simulink model "l8_ver100"  (Simulink 7.9)
settings: Solver=FixedStepDiscrete  SolverMode=SingleTasking  StartTime=0.0  StopTime=10  RelTol=1e-3
undefined symbols (not defined in the file): sfp
libraries: xbsIndex_r4 x17119, xps_library x133, casper_library_flow_control x73, casper_library_misc x61, dspsigops x32
(part 5 of 159)

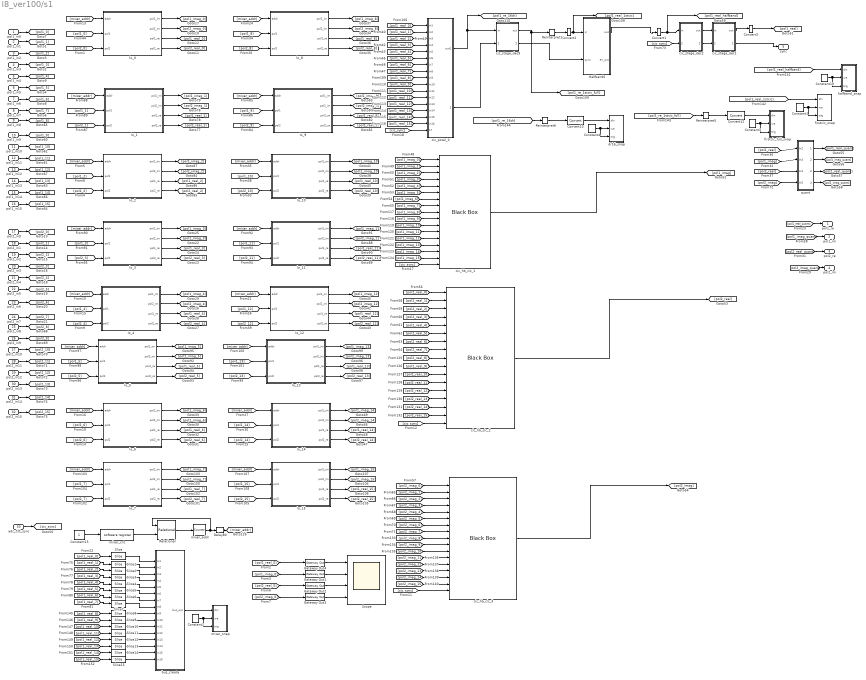
== system l8_ver100/s1 ==
  pol1_in0 : Inport
  pol1_in1 : Inport  Port=2
  pol1_in2 : Inport  Port=3
  pol1_in3 : Inport  Port=4
  pol1_in4 : Inport  Port=5
  pol1_in5 : Inport  Port=6
  pol1_in6 : Inport  Port=7
  pol1_in7 : Inport  Port=8
  pol1_in8 : Inport  Port=9
  pol1_in9 : Inport  Port=10
  pol1_in10 : Inport  Port=11
  pol1_in11 : Inport  Port=12
  pol1_in12 : Inport  Port=13
  pol1_in13 : Inport  Port=14
  pol1_in14 : Inport  Port=15
  pol1_in15 : Inport  Port=16
  pol2_in0 : Inport  Port=17
  pol2_in1 : Inport  Port=18
  pol2_in2 : Inport  Port=19
  pol2_in3 : Inport  Port=20
  pol2_in4 : Inport  Port=21
  pol2_in5 : Inport  Port=22
  pol2_in6 : Inport  Port=23
  pol2_in7 : Inport  Port=24
  pol2_in8 : Inport  Port=25
  pol2_in9 : Inport  Port=26
  pol2_in10 : Inport  Port=27
  pol2_in11 : Inport  Port=28
  pol2_in12 : Inport  Port=29
  pol2_in13 : Inport  Port=30
  pol2_in14 : Inport  Port=31
  pol2_in15 : Inport  Port=32
  adc_CIC_sync : Inport  Port=33
  Constant{1..4,19} (5 alike) : xbsIndex_r4/Constant  const=1  gui_display_data_type=Boolean  arith_type=Boolean  n_bits=32  bin_pt=0  preci_type=Single  exp_width=8  frac_width=24  explicit_period=on  period=1  equ=P=C  opselect=C  inp2=PCIN>>17  opr=+  inp1=P  carry=CIN  dbl_ovrd=off  block_type=constant
      settings: block_version=11.4
  Constant15 : Constant
  Convert1 : xbsIndex_r4/Convert  gui_display_data_type=Fixed-point  arith_type="Signed  (2's comp)"  n_bits=32  bin_pt=12  float_type=Single  exp_bits=8  fraction_bits=24  quantization=Truncate  overflow=Wrap  en=off  latency=1  dbl_ovrd=off  pipeline=off  block_type=convert
  Convert12 : xbsIndex_r4/Convert  gui_display_data_type=Fixed-point  arith_type="Signed  (2's comp)"  n_bits=64  bin_pt=0  float_type=Single  exp_bits=8  fraction_bits=24  quantization=Truncate  overflow=Wrap  en=off  latency=1  dbl_ovrd=off  pipeline=off  block_type=convert
  Convert13 : xbsIndex_r4/Convert  gui_display_data_type=Fixed-point  arith_type="Signed  (2's comp)"  n_bits=64  bin_pt=0  float_type=Single  exp_bits=8  fraction_bits=24  quantization=Truncate  overflow=Wrap  en=off  latency=1  dbl_ovrd=off  pipeline=off  block_type=convert
  Convert2 : xbsIndex_r4/Convert  gui_display_data_type=Fixed-point  arith_type="Signed  (2's comp)"  n_bits=32  bin_pt=18  float_type=Single  exp_bits=8  fraction_bits=24  quantization=Truncate  overflow=Wrap  en=off  latency=1  dbl_ovrd=off  pipeline=off  block_type=convert
  Convert3 : xbsIndex_r4/Convert  gui_display_data_type=Fixed-point  arith_type="Signed  (2's comp)"  n_bits=32  bin_pt=6  float_type=Single  exp_bits=8  fraction_bits=24  quantization=Truncate  overflow=Wrap  en=off  latency=1  dbl_ovrd=off  pipeline=off  block_type=convert
  Delay80 : xbsIndex_r4/Delay  rst=off  infoeditControl=Selection of Reset will increase slice count due to use of real FFs and instead of SRLs  en=off  latency=2  dbl_ovrd=off  reg_retiming=on  block_type=delay
      settings: block_version=11.4
  Gateway Out{1..4} (4 alike) : xbsIndex_r4/Gateway Out  inherit_from_input=off  hdl_port=on  timing_constraint=None  locs_specified=off  LOCs={}  UseAsDAC=off  DACChannel='1'  block_type=gatewayout
      settings: infoedit="Gateway out block.  Converts Xilinx fixed point or floating-point type inputs into ouputs of type Simulink integer, double, or fixed point.<br><br>Hardware notes:  In hardware these blocks become top level output ports or are discarded, depending on how they are configured."
  Halfband0 : Subsystem  AncestorBlock=gbt_library/Halfband  mask(Halfband FIR){mult_latency=3, add_latency=1, hold_period=2, passband=0.4, fir_len=7}
      mask prompts: mult_latency "Multiplication latency"; add_latency "Add latency"; hold_period "Hold Period"; passband "Width of Halfband passband (<0.5)"; fir_len "FIR Length (odd) [Number of Taps will be: 2*fir_len+1]"
      mask help: A halfband FIR for low rate computation.
      mask init: same as l8_ver100/s0/Halfband0
      settings: AttributesFormatString=|
      | 15-tap FIR filter (9 nonzero tap)
      |  hold period: 2
      |  mult latency: 3
      |  add latency: 1
  Reinterpret1 : xbsIndex_r4/Reinterpret  force_arith_type=off  arith_type="Signed  (2's comp)"  force_bin_pt=on  bin_pt=30  block_type=reinterpret
  Reinterpret5 : xbsIndex_r4/Reinterpret  force_arith_type=off  arith_type="Signed  (2's comp)"  force_bin_pt=on  bin_pt=0  block_type=reinterpret
  Reinterpret6 : xbsIndex_r4/Reinterpret  force_arith_type=off  arith_type="Signed  (2's comp)"  force_bin_pt=on  bin_pt=0  block_type=reinterpret
  Relational : xbsIndex_r4/Relational  mode=a=b  en=off  latency=1  dbl_ovrd=off  block_type=relational
  Scope : Scope  NumInputPorts=4
      settings: YMin=-0.0075~-1~-0.0075~-1  YMax=0.0075~1~0.0075~1
  Slice{,1..15} (16 alike) : xbsIndex_r4/Slice  nbits=8  boolean_output=off  mode=Upper Bit Location + Width  bit1=0  base1=MSB of Input  bit0=0  base0=LSB of Input  dbl_ovrd=off  block_type=slice
  bus_create : Subsystem  AncestorBlock=casper_library_flow_control/bus_create  mask(bus_create){inputNum=16}
      mask prompts: inputNum "Number of inputs:"
      mask init:
      | bus_create_init(gcb,...
      |     'inputNum', inputNum);
  cic_hb_cic_1 : xbsIndex_r4/Black Box  init_code=cichbcic_core_config  sim_method=Inactive  verbose=off  block_type=blackbox2
  cic_hb_cic_2 : xbsIndex_r4/Black Box  init_code=cichbcic_core_config  sim_method=Inactive  verbose=off  block_type=blackbox2
  cic_hb_cic_3 : xbsIndex_r4/Black Box  init_code=cichbcic_core_config  sim_method=Inactive  verbose=off  block_type=blackbox2
  cic_pow2_0 : Subsystem  AncestorBlock=gbt_library/cic_pow2  mask(CIC (pow2)){n_stages=4, dec_rate=16, input_clk_period=1, n_inputs=16, add_latency=1, n_bits=24, bin_pt=22}
      mask prompts: n_stages "CIC filter Order"; dec_rate "Decimation rate"; input_clk_period "Input Clock Period"; n_inputs "Number of inputs"; add_latency "Add latency"; n_bits "Input bit width"; bin_pt "Binary point"
      mask help:
      | A non-recursive CIC filter with parallel inputs.
      | Supports decimation rate of power of 2.
      | A multi-stage implementation with each stage of decimation rate 2.
      mask init: same as l8_ver100/s0/cic_pow2_0
      settings: AttributesFormatString=|
      | dec_rate = 16
      | Order = 4
  cic_stage_dec1 : Subsystem  AncestorBlock=gbt_library/cic_stage_dec2  mask(CIC stage (dec2)){n_stages=3, recursive=off, dec_rate=2, n_inputs=1, input_clk_period=1, input_period=4, add_latency=2, n_bits=35, bin_pt=12}
      mask prompts: n_stages "CIC filter Order"; recursive "Recursive?"; dec_rate "Decimation rate"; n_inputs "Number of Inputs"; input_clk_period "Input Clock period (Actual)"; input_period "Effective Input Sampling Period"; add_latency "Add latency"; n_bits "Input bit width"; bin_pt "Binary point"
      mask help:
      | A single stage of CIC filter with even number of parallel inputs.
      | Only supports decimation rate = 2.
      | If n_inputs > 1, number of outputs = n_inputs/2.
      | Non-recursive.
      |
      | Clock period:
      | input_clk_period is the actual sampling period of incoming data stream.
      | input_period is the effective (or expected) sampling period of incoming data stream.
      | E.g. For incoming data stream X[n] = [1& 1 2 2 3 3 4 4 5 5 ...], the input_clk_period is 1 and the input_period is 2.
      mask init: same as l8_ver100/s0/cic_stage_dec1
      settings: AttributesFormatString=|
      | nonrecursive
      | 35_12_in
      | clock(actualy/effective): 1/4
      mask CallbackString:
      | ||n=get_param(gcb,'dec_rate');
      | if ~isempty(n)
      |     n = eval(n);
      |     if ~isprime(n) && n~=1
      |         errordlg('Only supports decimation rate that is a prime number or 1');
      |         set_param(gcb,'dec_rate','');
      |     end
      | end||||||
  cic_stage_dec2 : Subsystem  AncestorBlock=gbt_library/cic_stage_dec2  mask(CIC stage (dec2)){n_stages=3, recursive=off, dec_rate=2, n_inputs=1, input_clk_period=1, input_period=2, add_latency=2, n_bits=32, bin_pt=12}
      mask prompts: n_stages "CIC filter Order"; recursive "Recursive?"; dec_rate "Decimation rate"; n_inputs "Number of Inputs"; input_clk_period "Input Clock period (Actual)"; input_period "Effective Input Sampling Period"; add_latency "Add latency"; n_bits "Input bit width"; bin_pt "Binary point"
      mask help:
      | A single stage of CIC filter with even number of parallel inputs.
      | Only supports decimation rate = 2.
      | If n_inputs > 1, number of outputs = n_inputs/2.
      | Non-recursive.
      |
      | Clock period:
      | input_clk_period is the actual sampling period of incoming data stream.
      | input_period is the effective (or expected) sampling period of incoming data stream.
      | E.g. For incoming data stream X[n] = [1& 1 2 2 3 3 4 4 5 5 ...], the input_clk_period is 1 and the input_period is 2.
      mask init: same as l8_ver100/s0/cic_stage_dec1
      settings: AttributesFormatString=|
      | nonrecursive
      | 32_12_in
      | clock(actualy/effective): 1/2
      mask CallbackString:
      | ||n=get_param(gcb,'dec_rate');
      | if ~isempty(n)
      |     n = eval(n);
      |     if ~isprime(n) && n~=1
      |         errordlg('Only supports decimation rate that is a prime number or 1');
      |         set_param(gcb,'dec_rate','');
      |     end
      | end||||||
  cic_stage_dec9 : Subsystem  AncestorBlock=gbt_library/cic_stage_dec2  mask(CIC stage (dec2)){n_stages=4, recursive=off, dec_rate=2, n_inputs=1, input_clk_period=1, input_period=1, add_latency=2, n_bits=40, bin_pt=22}
      mask prompts: n_stages "CIC filter Order"; recursive "Recursive?"; dec_rate "Decimation rate"; n_inputs "Number of Inputs"; input_clk_period "Input Clock period (Actual)"; input_period "Effective Input Sampling Period"; add_latency "Add latency"; n_bits "Input bit width"; bin_pt "Binary point"
      mask help:
      | A single stage of CIC filter with even number of parallel inputs.
      | Only supports decimation rate = 2.
      | If n_inputs > 1, number of outputs = n_inputs/2.
      | Non-recursive.
      |
      | Clock period:
      | input_clk_period is the actual sampling period of incoming data stream.
      | input_period is the effective (or expected) sampling period of incoming data stream.
      | E.g. For incoming data stream X[n] = [1& 1 2 2 3 3 4 4 5 5 ...], the input_clk_period is 1 and the input_period is 2.
      mask init: same as l8_ver100/s0/cic_stage_dec1
      settings: AttributesFormatString=|
      | nonrecursive
      | 40_22_in
      | clock(actualy/effective): 1/1
      mask CallbackString:
      | ||n=get_param(gcb,'dec_rate');
      | if ~isempty(n)
      |     n = eval(n);
      |     if ~isprime(n) && n~=1
      |         errordlg('Only supports decimation rate that is a prime number or 1');
      |         set_param(gcb,'dec_rate','');
      |     end
      | end||||||
  dr16_snap : Subsystem  AncestorBlock=casper_library_scopes/snapshot  mask(snapshot){storage=bram, dram_dimm=1, dram_clock=200, nsamples=12, data_width=64, offset=off, circap=off, value=off, use_dsp48=on}
      mask prompts: storage "Storage medium"; dram_dimm "DRAM dimm"; dram_clock "DRAM clock rate"; nsamples "Number of Samples (2^?)"; data_width "Data width"; offset "Start delay support"; circap "Circular capture support"; value "Extra value capture support"; use_dsp48 "Use DSP48s to implement counters"
      mask help:
      | Standard snap block, but with the following optional extras;
      |  1) Optionally delay the fabric trigger by a user-specified number of valids.
      |  2) Capture continuously after a trigger until a stop pulse is received. Data is captured into BRAM continuously, with old data being overwritten until a stop command is received. Then stop capturing when memory is filled. This enables you to get some data before and some data after the stop pulse. Use the 'tr_en_cnt' register to determine where you are in your vector. 'Addr' register's MSb now indicates 'busy capturing'.
      |  3) Optionally capture a value on an input port to a register at the same time as the first data sample.
      |  4) Implement counters using DSP48Es to save resources.
      mask init: same as l8_ver100/adcsnap0
      mask StyleString: popup(bram|dram),popup(1|2|3|4),popup(200|250|266|300|333),edit,popup(8|16|32|64|128),checkbox,checkbox,checkbox,checkbox
      mask CallbackString: snapshot_callback();||||||||
  firstcic_full_snap : Subsystem  AncestorBlock=casper_library_scopes/snapshot  mask(snapshot){storage=bram, dram_dimm=1, dram_clock=200, nsamples=12, data_width=64, offset=off, circap=off, value=off, use_dsp48=on}
      mask prompts: storage "Storage medium"; dram_dimm "DRAM dimm"; dram_clock "DRAM clock rate"; nsamples "Number of Samples (2^?)"; data_width "Data width"; offset "Start delay support"; circap "Circular capture support"; value "Extra value capture support"; use_dsp48 "Use DSP48s to implement counters"
      mask help:
      | Standard snap block, but with the following optional extras;
      |  1) Optionally delay the fabric trigger by a user-specified number of valids.
      |  2) Capture continuously after a trigger until a stop pulse is received. Data is captured into BRAM continuously, with old data being overwritten until a stop command is received. Then stop capturing when memory is filled. This enables you to get some data before and some data after the stop pulse. Use the 'tr_en_cnt' register to determine where you are in your vector. 'Addr' register's MSb now indicates 'busy capturing'.
      |  3) Optionally capture a value on an input port to a register at the same time as the first data sample.
      |  4) Implement counters using DSP48Es to save resources.
      mask init: same as l8_ver100/adcsnap0
      mask StyleString: popup(bram|dram),popup(1|2|3|4),popup(200|250|266|300|333),edit,popup(8|16|32|64|128),checkbox,checkbox,checkbox,checkbox
      mask CallbackString: snapshot_callback();||||||||
  firstcic_snap : Subsystem  AncestorBlock=casper_library_scopes/snapshot  mask(snapshot){storage=bram, dram_dimm=1, dram_clock=200, nsamples=12, data_width=32, offset=off, circap=off, value=off, use_dsp48=on}
      mask prompts: storage "Storage medium"; dram_dimm "DRAM dimm"; dram_clock "DRAM clock rate"; nsamples "Number of Samples (2^?)"; data_width "Data width"; offset "Start delay support"; circap "Circular capture support"; value "Extra value capture support"; use_dsp48 "Use DSP48s to implement counters"
      mask help:
      | Standard snap block, but with the following optional extras;
      |  1) Optionally delay the fabric trigger by a user-specified number of valids.
      |  2) Capture continuously after a trigger until a stop pulse is received. Data is captured into BRAM continuously, with old data being overwritten until a stop command is received. Then stop capturing when memory is filled. This enables you to get some data before and some data after the stop pulse. Use the 'tr_en_cnt' register to determine where you are in your vector. 'Addr' register's MSb now indicates 'busy capturing'.
      |  3) Optionally capture a value on an input port to a register at the same time as the first data sample.
      |  4) Implement counters using DSP48Es to save resources.
      mask init: same as l8_ver100/adcsnap0
      mask StyleString: popup(bram|dram),popup(1|2|3|4),popup(200|250|266|300|333),edit,popup(8|16|32|64|128),checkbox,checkbox,checkbox,checkbox
      mask CallbackString: snapshot_callback();||||||||
  halfband_snap : Subsystem  AncestorBlock=casper_library_scopes/snapshot  mask(snapshot){storage=bram, dram_dimm=1, dram_clock=200, nsamples=12, data_width=32, offset=off, circap=off, value=off, use_dsp48=on}
      mask prompts: storage "Storage medium"; dram_dimm "DRAM dimm"; dram_clock "DRAM clock rate"; nsamples "Number of Samples (2^?)"; data_width "Data width"; offset "Start delay support"; circap "Circular capture support"; value "Extra value capture support"; use_dsp48 "Use DSP48s to implement counters"
      mask help:
      | Standard snap block, but with the following optional extras;
      |  1) Optionally delay the fabric trigger by a user-specified number of valids.
      |  2) Capture continuously after a trigger until a stop pulse is received. Data is captured into BRAM continuously, with old data being overwritten until a stop command is received. Then stop capturing when memory is filled. This enables you to get some data before and some data after the stop pulse. Use the 'tr_en_cnt' register to determine where you are in your vector. 'Addr' register's MSb now indicates 'busy capturing'.
      |  3) Optionally capture a value on an input port to a register at the same time as the first data sample.
      |  4) Implement counters using DSP48Es to save resources.
      mask init: same as l8_ver100/adcsnap0
      mask StyleString: popup(bram|dram),popup(1|2|3|4),popup(200|250|266|300|333),edit,popup(8|16|32|64|128),checkbox,checkbox,checkbox,checkbox
      mask CallbackString: snapshot_callback();||||||||
  lo_0 : Subsystem  AncestorBlock=gbt_library/complex_mix_lo
  lo_1 : Subsystem  AncestorBlock=gbt_library/complex_mix_lo
  lo_10 : Subsystem  AncestorBlock=gbt_library/complex_mix_lo
  lo_11 : Subsystem  AncestorBlock=gbt_library/complex_mix_lo
  lo_12 : Subsystem  AncestorBlock=gbt_library/complex_mix_lo
  lo_13 : Subsystem  AncestorBlock=gbt_library/complex_mix_lo
  lo_14 : Subsystem  AncestorBlock=gbt_library/complex_mix_lo
  lo_15 : Subsystem  AncestorBlock=gbt_library/complex_mix_lo
  lo_2 : Subsystem  AncestorBlock=gbt_library/complex_mix_lo
  lo_3 : Subsystem  AncestorBlock=gbt_library/complex_mix_lo
  lo_4 : Subsystem  AncestorBlock=gbt_library/complex_mix_lo
  lo_5 : Subsystem  AncestorBlock=gbt_library/complex_mix_lo
  lo_6 : Subsystem  AncestorBlock=gbt_library/complex_mix_lo
  lo_7 : Subsystem  AncestorBlock=gbt_library/complex_mix_lo
  lo_8 : Subsystem  AncestorBlock=gbt_library/complex_mix_lo
  lo_9 : Subsystem  AncestorBlock=gbt_library/complex_mix_lo
  mixer_addr : xbsIndex_r4/Counter  cnt_type=Free Running  cnt_to=Inf  operation=Up  start_count=0  cnt_by_val=1  arith_type=Unsigned  n_bits=11  bin_pt=0  load_pin=off  rst=on  en=off  explicit_period=on  period=1  dbl_ovrd=off  use_behavioral_HDL=off  implementation=Fabric  block_type=counter
      settings: block_version=11.4
  mixer_cnt : xps_library/software register  Tag=xps:sw_reg  io_dir=From Processor  arith_type=Unsigned  bitwidth=32  bin_pt=0  sample_period=1  gw_name=l8_ver100_s1_mixer_cnt_user_data_out
      link override newmode13_mixer_cnt_user_data_out: arith_type=Unsigned  n_bits=32  bin_pt=0  period=1
  mixer_snap : Subsystem  AncestorBlock=casper_library_scopes/snapshot  mask(snapshot){storage=bram, dram_dimm=1, dram_clock=200, nsamples=12, data_width=128, offset=off, circap=off, value=off, use_dsp48=on}
      mask prompts: storage "Storage medium"; dram_dimm "DRAM dimm"; dram_clock "DRAM clock rate"; nsamples "Number of Samples (2^?)"; data_width "Data width"; offset "Start delay support"; circap "Circular capture support"; value "Extra value capture support"; use_dsp48 "Use DSP48s to implement counters"
      mask help:
      | Standard snap block, but with the following optional extras;
      |  1) Optionally delay the fabric trigger by a user-specified number of valids.
      |  2) Capture continuously after a trigger until a stop pulse is received. Data is captured into BRAM continuously, with old data being overwritten until a stop command is received. Then stop capturing when memory is filled. This enables you to get some data before and some data after the stop pulse. Use the 'tr_en_cnt' register to determine where you are in your vector. 'Addr' register's MSb now indicates 'busy capturing'.
      |  3) Optionally capture a value on an input port to a register at the same time as the first data sample.
      |  4) Implement counters using DSP48Es to save resources.
      mask init: same as l8_ver100/adcsnap0
      mask StyleString: popup(bram|dram),popup(1|2|3|4),popup(200|250|266|300|333),edit,popup(8|16|32|64|128),checkbox,checkbox,checkbox,checkbox
      mask CallbackString: snapshot_callback();||||||||
  quant : Subsystem
  pol1_re : Outport
  pol1_im : Outport  Port=2
  pol2_re : Outport  Port=3
  pol2_im : Outport  Port=4
  sync : Outport  Port=5
  wires:
  pol1_in0/1 -> [pol1_0]
  pol1_in1/1 -> [pol1_1]
  pol1_in2/1 -> [pol1_2]
  pol1_in3/1 -> [pol1_3]
  pol1_in4/1 -> [pol1_4]
  pol1_in5/1 -> [pol1_5]
  pol1_in6/1 -> [pol1_6]
  pol1_in7/1 -> [pol1_7]
  pol2_in0/1 -> [pol2_0]
  pol2_in1/1 -> [pol2_1]
  pol2_in2/1 -> [pol2_2]
  pol2_in3/1 -> [pol2_3]
  pol2_in4/1 -> [pol2_4]
  pol2_in5/1 -> [pol2_5]
  pol2_in6/1 -> [pol2_6]
  pol2_in7/1 -> [pol2_7]
  [mixer_addr] -> lo_2/1(addr)
  [mixer_addr] -> lo_4/1(addr)
  [mixer_addr] -> lo_6/1(addr)
  lo_8/1(pol1_im) -> [pol1_imag_8]
  lo_8/2(pol2_im) -> [pol2_imag_8]
  lo_8/3(pol1_re) -> [pol1_real_8]
  lo_8/4(pol2_re) -> [pol2_real_8]
  [mixer_addr] -> lo_8/1(addr)
  [pol2_8] -> lo_8/3(pol2)
  [pol1_8] -> lo_8/2(pol1)
  [mixer_addr] -> lo_0/1(addr)
  [pol1_0] -> lo_0/2(pol1)
  [pol2_0] -> lo_0/3(pol2)
  lo_0/1(pol1_im) -> [pol1_imag_0]
  lo_0/2(pol2_im) -> [pol2_imag_0]
  lo_0/3(pol1_re) -> [pol1_real_0]
  lo_0/4(pol2_re) -> [pol2_real_0]
  [pol1_2] -> lo_2/2(pol1)
  [pol2_2] -> lo_2/3(pol2)
  [pol1_4] -> lo_4/2(pol1)
  [pol2_4] -> lo_4/3(pol2)
  [pol1_6] -> lo_6/2(pol1)
  [pol2_6] -> lo_6/3(pol2)
  lo_6/1(pol1_im) -> [pol1_imag_6]
  lo_6/2(pol2_im) -> [pol2_imag_6]
  lo_6/3(pol1_re) -> [pol1_real_6]
  lo_6/4(pol2_re) -> [pol2_real_6]
  [mixer_addr] -> lo_10/1(addr)
  [pol1_10] -> lo_10/2(pol1)
  [pol2_10] -> lo_10/3(pol2)
  lo_10/1(pol1_im) -> [pol1_imag_10]
  lo_10/2(pol2_im) -> [pol2_imag_10]
  lo_10/3(pol1_re) -> [pol1_real_10]
  lo_10/4(pol2_re) -> [pol2_real_10]
  [mixer_addr] -> lo_12/1(addr)
  [pol1_12] -> lo_12/2(pol1)
  [pol2_12] -> lo_12/3(pol2)
  lo_12/1(pol1_im) -> [pol1_imag_12]
  lo_12/2(pol2_im) -> [pol2_imag_12]
  lo_12/3(pol1_re) -> [pol1_real_12]
  lo_12/4(pol2_re) -> [pol2_real_12]
  [mixer_addr] -> lo_14/1(addr)
  [pol1_14] -> lo_14/2(pol1)
  [pol2_14] -> lo_14/3(pol2)
  lo_14/1(pol1_im) -> [pol1_imag_14]
  lo_14/2(pol2_im) -> [pol2_imag_14]
  lo_14/3(pol1_re) -> [pol1_real_14]
  lo_14/4(pol2_re) -> [pol2_real_14]
  lo_4/1(pol1_im) -> [pol1_imag_4]
  lo_4/2(pol2_im) -> [pol2_imag_4]
  lo_4/3(pol1_re) -> [pol1_real_4]
  lo_4/4(pol2_re) -> [pol2_real_4]
  [pol1_real_0] -> Gateway Out4/1
  [pol1_imag_0] -> Gateway Out1/1
  [pol2_real_0] -> Gateway Out2/1
  [pol2_imag_0] -> Gateway Out3/1
  Gateway Out4/1 -> Scope/1
  Gateway Out1/1 -> Scope/2
  Gateway Out2/1 -> Scope/3
  Gateway Out3/1 -> Scope/4
  adc_CIC_sync/1 -> [cic_sync]
  [pol1_real_quant] -> pol1_re/1
  cic_stage_dec2/2(sync_out) -> cic_stage_dec1/2(sync_in)
  cic_stage_dec2/1(out) -> cic_stage_dec1/1(in)
  Halfband0/1(out) -> Convert1/1
  [cic_sync] -> cic_stage_dec2/2(sync_in)
  Convert2/1 -> Halfband0/1(in), [pol1_real_1stcic]
  Convert1/1 -> cic_stage_dec2/1(in), [pol1_real_halfband]
  Convert3/1 -> [pol1_real]
  [pol1_imag_quant] -> pol1_im/1
  quant/1(Out1) -> [pol1_real_quant]
  quant/2(Out2) -> [pol1_imag_quant]
  [pol1_real] -> quant/1(In2)
  [pol1_imag] -> quant/2(In3)
  [pol2_real] -> quant/3(In4)
  [pol2_imag] -> quant/4(In5)
  quant/3(Out3) -> [pol2_real_quant]
  quant/4(Out4) -> [pol2_imag_quant]
  [pol2_real_quant] -> pol2_re/1
  [pol2_imag_quant] -> pol2_im/1
  pol1_in8/1 -> [pol1_8]
  pol1_in9/1 -> [pol1_9]
  pol1_in10/1 -> [pol1_10]
  pol1_in11/1 -> [pol1_11]
  pol1_in12/1 -> [pol1_12]
  pol1_in13/1 -> [pol1_13]
  pol1_in14/1 -> [pol1_14]
  pol1_in15/1 -> [pol1_15]
  pol2_in8/1 -> [pol2_8]
  pol2_in9/1 -> [pol2_9]
  pol2_in10/1 -> [pol2_10]
  pol2_in11/1 -> [pol2_11]
  pol2_in12/1 -> [pol2_12]
  pol2_in13/1 -> [pol2_13]
  pol2_in14/1 -> [pol2_14]
  pol2_in15/1 -> [pol2_15]
  [mixer_addr] -> lo_1/1(addr)
  [pol1_1] -> lo_1/2(pol1)
  [pol2_1] -> lo_1/3(pol2)
  lo_1/1(pol1_im) -> [pol1_imag_1]
  lo_1/2(pol2_im) -> [pol2_imag_1]
  lo_1/3(pol1_re) -> [pol1_real_1]
  lo_1/4(pol2_re) -> [pol2_real_1]
  [mixer_addr] -> lo_9/1(addr)
  [pol1_9] -> lo_9/2(pol1)
  [pol2_9] -> lo_9/3(pol2)
  lo_9/1(pol1_im) -> [pol1_imag_9]
  lo_9/2(pol2_im) -> [pol2_imag_9]
  lo_9/3(pol1_re) -> [pol1_real_9]
  lo_9/4(pol2_re) -> [pol2_real_9]
  [mixer_addr] -> lo_3/1(addr)
  [pol1_3] -> lo_3/2(pol1)
  [pol2_3] -> lo_3/3(pol2)
  [mixer_addr] -> lo_11/1(addr)
  [pol1_11] -> lo_11/2(pol1)
  [pol2_11] -> lo_11/3(pol2)
  lo_11/1(pol1_im) -> [pol1_imag_11]
  lo_11/2(pol2_im) -> [pol2_imag_11]
  lo_11/3(pol1_re) -> [pol1_real_11]
  lo_11/4(pol2_re) -> [pol2_real_11]
  [mixer_addr] -> lo_5/1(addr)
  [pol1_5] -> lo_5/2(pol1)
  [pol2_5] -> lo_5/3(pol2)
  lo_5/1(pol1_im) -> [pol1_imag_5]
  lo_5/2(pol2_im) -> [pol2_imag_5]
  lo_5/3(pol1_re) -> [pol1_real_5]
  lo_5/4(pol2_re) -> [pol2_real_5]
  [mixer_addr] -> lo_13/1(addr)
  [pol1_13] -> lo_13/2(pol1)
  [pol2_13] -> lo_13/3(pol2)
  lo_13/1(pol1_im) -> [pol1_imag_13]
  lo_13/2(pol2_im) -> [pol2_imag_13]
  lo_13/3(pol1_re) -> [pol1_real_13]
  lo_13/4(pol2_re) -> [pol2_real_13]
  [mixer_addr] -> lo_7/1(addr)
  [pol1_7] -> lo_7/2(pol1)
  [pol2_7] -> lo_7/3(pol2)
  lo_7/1(pol1_im) -> [pol1_imag_7]
  lo_7/2(pol2_im) -> [pol2_imag_7]
  lo_7/3(pol1_re) -> [pol1_real_7]
  lo_7/4(pol2_re) -> [pol2_real_7]
  [mixer_addr] -> lo_15/1(addr)
  [pol1_15] -> lo_15/2(pol1)
  [pol2_15] -> lo_15/3(pol2)
  lo_15/1(pol1_im) -> [pol1_imag_15]
  lo_15/2(pol2_im) -> [pol2_imag_15]
  lo_15/3(pol1_re) -> [pol1_real_15]
  lo_15/4(pol2_re) -> [pol2_real_15]
  lo_2/2(pol2_im) -> [pol2_imag_2]
  lo_2/1(pol1_im) -> [pol1_imag_2]
  lo_2/3(pol1_re) -> [pol1_real_2]
  lo_2/4(pol2_re) -> [pol2_real_2]
  lo_3/1(pol1_im) -> [pol1_imag_3]
  lo_3/2(pol2_im) -> [pol2_imag_3]
  lo_3/3(pol1_re) -> [pol1_real_3]
  lo_3/4(pol2_re) -> [pol2_real_3]
  [cic_sync] -> cic_pow2_0/17(sync_in)
  [pol1_real_0] -> cic_pow2_0/1(in1)
  [pol1_real_1] -> cic_pow2_0/2(in2)
  [pol1_real_2] -> cic_pow2_0/3(in3)
  [pol1_real_3] -> cic_pow2_0/4(in4)
  [pol1_real_4] -> cic_pow2_0/5(in5)
  [pol1_real_5] -> cic_pow2_0/6(in6)
  [pol1_real_6] -> cic_pow2_0/7(in7)
  [pol1_real_7] -> cic_pow2_0/8(in8)
  [pol1_real_8] -> cic_pow2_0/9(in9)
  [pol1_real_9] -> cic_pow2_0/10(in10)
  [pol1_real_10] -> cic_pow2_0/11(in11)
  [pol1_real_11] -> cic_pow2_0/12(in12)
  [pol1_real_12] -> cic_pow2_0/13(in13)
  [pol1_real_13] -> cic_pow2_0/14(in14)
  [pol1_real_14] -> cic_pow2_0/15(in15)
  [pol1_real_15] -> cic_pow2_0/16(in16)
  [cic_sync] -> cic_hb_cic_1/17
  [pol1_imag_0] -> cic_hb_cic_1/1
  [pol1_imag_1] -> cic_hb_cic_1/2
  [pol1_imag_2] -> cic_hb_cic_1/3
  [pol1_imag_3] -> cic_hb_cic_1/4
  [pol1_imag_4] -> cic_hb_cic_1/5
  [pol1_imag_5] -> cic_hb_cic_1/6
  [pol1_imag_6] -> cic_hb_cic_1/7
  [pol1_imag_7] -> cic_hb_cic_1/8
  [pol1_imag_8] -> cic_hb_cic_1/9
  [pol1_imag_9] -> cic_hb_cic_1/10
  [pol1_imag_10] -> cic_hb_cic_1/11
  [pol1_imag_11] -> cic_hb_cic_1/12
  [pol1_imag_12] -> cic_hb_cic_1/13
  [pol1_imag_13] -> cic_hb_cic_1/14
  [pol1_imag_14] -> cic_hb_cic_1/15
  [pol1_imag_15] -> cic_hb_cic_1/16
  cic_stage_dec1/1(out) -> Convert3/1
  cic_stage_dec1/2(sync_out) -> sync/1
  Constant19/1 -> halfband_snap/3(trig), halfband_snap/2(we)
  [pol1_real_halfband] -> halfband_snap/1(din)
  Constant1/1 -> firstcic_snap/3(trig), firstcic_snap/2(we)
  [pol1_real_1stcic] -> firstcic_snap/1(din)
  Reinterpret1/1 -> Convert2/1
  Constant2/1 -> mixer_snap/2(we), mixer_snap/3(trig)
  bus_create/1(bus_out) -> mixer_snap/1(din)
  [pol1_real_0] -> Slice/1
  [pol1_real_1] -> Slice1/1
  [pol1_real_2] -> Slice2/1
  [pol1_real_3] -> Slice3/1
  [pol1_real_4] -> Slice4/1
  [pol1_real_5] -> Slice5/1
  [pol1_real_6] -> Slice6/1
  [pol1_real_7] -> Slice7/1
  [pol1_real_8] -> Slice8/1
  [pol1_real_9] -> Slice9/1
  [pol1_real_10] -> Slice10/1
  [pol1_real_11] -> Slice11/1
  [pol1_real_12] -> Slice12/1
  [pol1_real_13] -> Slice13/1
  [pol1_real_14] -> Slice14/1
  [pol1_real_15] -> Slice15/1
  Slice/1 -> bus_create/1(in1)
  Slice1/1 -> bus_create/2(in2)
  Slice2/1 -> bus_create/3(in3)
  Slice3/1 -> bus_create/4(in4)
  Slice4/1 -> bus_create/5(in5)
  Slice5/1 -> bus_create/6(in6)
  Slice6/1 -> bus_create/7(in7)
  Slice7/1 -> bus_create/8(in8)
  Slice8/1 -> bus_create/9(in9)
  Slice9/1 -> bus_create/10(in10)
  Slice10/1 -> bus_create/11(in11)
  Slice11/1 -> bus_create/12(in12)
  Slice12/1 -> bus_create/13(in13)
  Slice13/1 -> bus_create/14(in14)
  Slice14/1 -> bus_create/15(in15)
  Slice15/1 -> bus_create/16(in16)
  Constant3/1 -> firstcic_full_snap/3(trig), firstcic_full_snap/2(we)
  [pol1_re_1stcic_full] -> Reinterpret5/1
  Reinterpret5/1 -> Convert12/1
  Convert12/1 -> firstcic_full_snap/1(din)
  cic_pow2_0/1(out1) -> cic_stage_dec9/1(in), [pol1_re_16dr]
  cic_pow2_0/2(sync_out) -> cic_stage_dec9/2(sync_in)
  cic_stage_dec9/1(out) -> Reinterpret1/1, [pol1_re_1stcic_full]
  cic_stage_dec9/2(sync_out) -> Halfband0/2(sync)
  Constant4/1 -> dr16_snap/3(trig), dr16_snap/2(we)
  [pol1_re_16dr] -> Reinterpret6/1
  Reinterpret6/1 -> Convert13/1
  Convert13/1 -> dr16_snap/1(din)
  mixer_addr/1 -> Relational/1, Delay80/1
  Delay80/1 -> [mixer_addr]
  Relational/1 -> mixer_addr/1
  mixer_cnt/1 -> Relational/2
  Constant15/1 -> mixer_cnt/1
  cic_hb_cic_1/1 -> [pol1_imag]
  [pol2_real_0] -> cic_hb_cic_2/1
  [pol2_real_1] -> cic_hb_cic_2/2
  [pol2_real_2] -> cic_hb_cic_2/3
  [pol2_real_3] -> cic_hb_cic_2/4
  [pol2_real_4] -> cic_hb_cic_2/5
  [pol2_real_5] -> cic_hb_cic_2/6
  [pol2_real_6] -> cic_hb_cic_2/7
  [pol2_real_7] -> cic_hb_cic_2/8
  [pol2_real_8] -> cic_hb_cic_2/9
  [pol2_real_9] -> cic_hb_cic_2/10
  [pol2_real_10] -> cic_hb_cic_2/11
  [pol2_real_11] -> cic_hb_cic_2/12
  [pol2_real_12] -> cic_hb_cic_2/13
  [pol2_real_13] -> cic_hb_cic_2/14
  [pol2_real_14] -> cic_hb_cic_2/15
  [pol2_real_15] -> cic_hb_cic_2/16
  [cic_sync] -> cic_hb_cic_2/17
  cic_hb_cic_2/1 -> [pol2_real]
  [pol2_imag_0] -> cic_hb_cic_3/1
  [pol2_imag_1] -> cic_hb_cic_3/2
  [pol2_imag_2] -> cic_hb_cic_3/3
  [pol2_imag_3] -> cic_hb_cic_3/4
  [pol2_imag_4] -> cic_hb_cic_3/5
  [pol2_imag_5] -> cic_hb_cic_3/6
  [pol2_imag_6] -> cic_hb_cic_3/7
  [pol2_imag_7] -> cic_hb_cic_3/8
  [pol2_imag_8] -> cic_hb_cic_3/9
  [pol2_imag_9] -> cic_hb_cic_3/10
  [pol2_imag_10] -> cic_hb_cic_3/11
  [pol2_imag_11] -> cic_hb_cic_3/12
  [pol2_imag_12] -> cic_hb_cic_3/13
  [pol2_imag_13] -> cic_hb_cic_3/14
  [pol2_imag_14] -> cic_hb_cic_3/15
  [pol2_imag_15] -> cic_hb_cic_3/16
  [cic_sync] -> cic_hb_cic_3/17
  cic_hb_cic_3/1 -> [pol2_imag]

== system l8_ver100/s1p1re_snap ==
  note: Generate stop
      | when in circular
      | capture mode
  note: Delay start
      | of capture
  note: ctrl:
      | bit0: Put a posedge here
      |         to enable a new capture.
      | bit1: ignore external trigger
      |         and trigger immediately.
      | bit2: ignore external write
      |         enable and capture on
      |         every FPGA clock.
      | bit3: continuously capture
      |         data until we get an
      |         external stop command.
  note: Offset in vector
      | from first element
      | captured, used
      | during circular capture
  note: Number of
      | bytes in
      | buffer and
      | done bit
  note: Number of valid
      | data samples to
      | skip after trigger
      | before starting
      | capture
  note: Value on vin
      | aligned with
      | first sample on
      | din captured
  din : Inport
  we : Inport  Port=2
  trig : Inport  Port=3
  add_del : xbsIndex_r4/Delay  rst=off  infoeditControl=Selection of Reset will increase slice count due to use of real FFs and instead of SRLs  en=off  latency=1  dbl_ovrd=off  reg_retiming=off  block_type=delay
  add_gen : Subsystem  AncestorBlock=casper_library_scopes/snapshot/add_gen  mask{counter_size=12+0+1, increment=1, nsamples=12, burst_size=0, use_dsp48=on}
      mask prompts: counter_size "Address counter size (2^?)"; increment "Address counter increment"; nsamples "Number of samples (2^?)"; burst_size "Burst size (2^?)"; use_dsp48 "dsp48 counter/s"
      mask init:
      | set_param(gcb,'LinkStatus','inactive');
      | if strcmp(use_dsp48,'on'),
      |     set_param([gcb,'/add_gen'],'implementation','DSP48');
      | else
      |     set_param([gcb,'/add_gen'],'implementation','Fabric');
      | end
  basic_ctrl : Subsystem  AncestorBlock=casper_library_scopes/snapshot/basic_ctrl  mask{dram=off, data_width=8}
      mask prompts: data_width "data width"
      mask init:
      | set_param(gcb,'LinkStatus','inactive');
      | set_param([gcb,'/dram_munge'], 'dram', dram);
  bram : Subsystem  Tag=xps:bram  AncestorBlock=xps_library/Shared BRAM  mask{arith_type=Unsigned, addr_width=12, data_width=8, data_bin_pt=0, init_vals=[0:2^10-1], sample_rate=1}
      mask prompts: arith_type "Output Data Type"; addr_width "Address width"; data_width "Data Width"; data_bin_pt "Data Binary Point"; init_vals "Initial 32-bit values (simulation only)"; sample_rate "Sample rate"
      mask init:
      | shared_bram_mask;
      mask StyleString: popup(Unsigned|Signed  (2's comp)),edit,popup(8|16|32|64|128),edit,edit,edit
  cast : xbsIndex_r4/Convert  gui_display_data_type=Fixed-point  arith_type=Unsigned  n_bits=8  bin_pt=0  float_type=Single  exp_bits=8  fraction_bits=24  quantization=Truncate  overflow=Wrap  en=off  latency=0  dbl_ovrd=off  pipeline=off  block_type=convert
  const0 : Constant  Value=0
  ctrl : xps_library/software register  Tag=xps:sw_reg  io_dir=From Processor  arith_type=Unsigned  bitwidth=32  bin_pt=0  sample_period=1  gw_name=l8_ver100_s1p1re_snap_ctrl_user_data_out
  dat_del : xbsIndex_r4/Delay  rst=off  infoeditControl=Selection of Reset will increase slice count due to use of real FFs and instead of SRLs  en=off  latency=1  dbl_ovrd=off  reg_retiming=off  block_type=delay
  g_tr_en_cnt : Terminator
  gbram : Terminator
  gstatus : Terminator
  gval : Terminator
  never : xbsIndex_r4/Constant  const=0  gui_display_data_type=Fixed-point  arith_type=Boolean  n_bits=16  bin_pt=14  preci_type=Single  exp_width=8  frac_width=24  explicit_period=on  period=1  equ=P=C  opselect=C  inp2=PCIN>>17  opr=+  inp1=P  carry=CIN  dbl_ovrd=off  block_type=constant
  ri : xbsIndex_r4/Reinterpret  force_arith_type=on  arith_type=Unsigned  force_bin_pt=on  bin_pt=0  block_type=reinterpret
  status : xps_library/software register  Tag=xps:sw_reg  io_dir=To Processor  arith_type=Unsigned  bitwidth=32  bin_pt=0  sample_period=1  gw_name=l8_ver100_s1p1re_snap_status_user_data_in
  vin_const : xbsIndex_r4/Constant  const=0  gui_display_data_type=Fixed-point  arith_type=Boolean  n_bits=16  bin_pt=14  preci_type=Single  exp_width=8  frac_width=24  explicit_period=on  period=1  equ=P=C  opselect=C  inp2=PCIN>>17  opr=+  inp1=P  carry=CIN  dbl_ovrd=off  block_type=constant
  we_del : xbsIndex_r4/Delay  rst=off  infoeditControl=Selection of Reset will increase slice count due to use of real FFs and instead of SRLs  en=off  latency=1  dbl_ovrd=off  reg_retiming=off  block_type=delay
  wires:
  din/1 -> ri/1
  ri/1 -> cast/1
  cast/1 -> basic_ctrl/2(din)
  we/1 -> basic_ctrl/3(we)
  trig/1 -> basic_ctrl/4(trig)
  never/1 -> basic_ctrl/6(stopi)
  const0/1 -> ctrl/1
  ctrl/1 -> basic_ctrl/5(ctrl)
  basic_ctrl/1(vout) -> add_gen/1(vin)
  basic_ctrl/2(dout) -> add_gen/2(din)
  basic_ctrl/3(we_o) -> add_gen/3(we)
  basic_ctrl/4(go) -> add_gen/4(go)
  basic_ctrl/5(stop) -> add_gen/5(cont)
  basic_ctrl/6(init) -> add_gen/6(init)
  add_gen/5(status) -> status/1
  status/1 -> gstatus/1
  vin_const/1 -> basic_ctrl/1(vin)
  add_gen/1(vout) -> gval/1
  add_gen/6(en_cnt) -> g_tr_en_cnt/1
  add_gen/2(add) -> add_del/1
  add_gen/3(dout) -> dat_del/1
  add_gen/4(we_o) -> we_del/1
  add_del/1 -> bram/1(addr)
  dat_del/1 -> bram/2(data_in)
  we_del/1 -> bram/3(we)
  bram/1(data_out) -> gbram/1
